simulink model "isi_corr"  (Simulink 7.0)
settings: Solver=ode45  StartTime=0.0  StopTime=500  RelTol=1e-3
undefined symbols (not defined in the file): bit_rev, pfb_coeff_gen_calc
libraries: xbsIndex_r4 x4180, casper_library x399, isi_corr_lib x8, xps_library x5, dspsigops x2, simulink x1
(part 1 of 45)
settings Model: Creator=wjm  LastModifiedBy=wjm

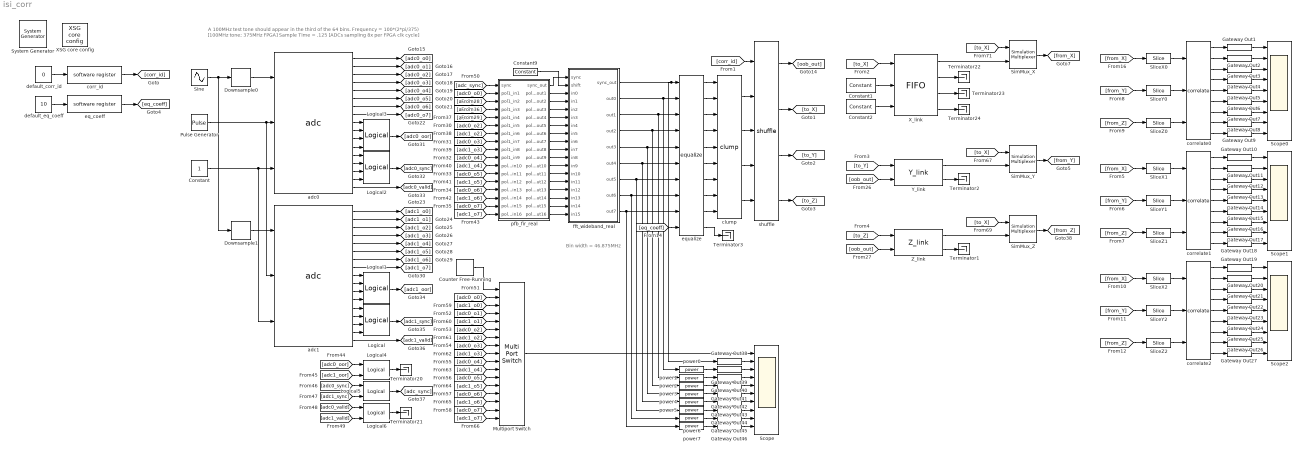
== system isi_corr ==
  note: A 100MHz test tone should appear in the third of the 64 bins.
      | Frequency = 100*(2*pi/375) [100MHz tone; 375MHz FPGA]
      | Sample Time = .125 [ADCs sampling 8x per FPGA clk cycle]
  note: Bin width = 46.875MHz
  " System Generator" : xbsIndex_r4/ System Generator  Tag=genX  SystemSampleTime=-1  xilinxfamily=virtex5  part=xc5vsx95t  speed=-1  package=ff1136  synthesis_tool=XST  clock_wrapper=Clock Enables  directory=./isi_corr/sysgen  testbench=off  simulink_period=1  sysclk_period=2.6667  dcm_input_clock_period=100  incr_netlist=off  trim_vbits=Everywhere in SubSystem  dbl_ovrd=According to Block Masks  core_generation=According to Block Masks  run_coregen=off  deprecated_control=off  eval_field=0  block_type=sysgen
      settings: block_version=10.1.1
  Constant : Constant  OutDataType=sfix(16)  OutScaling=2^0  SampleTime=1
  Constant1 : xbsIndex_r4/Constant  arith_type=Boolean  const=1  n_bits=1  bin_pt=0  explicit_period=on  period=1  equ=P=C  opselect=C  inp2=PCIN>>17  opr=+  inp1=P  carry=CIN  dbl_ovrd=off  block_type=constant
      settings: block_version=10.1.1
  Constant2 : xbsIndex_r4/Constant  arith_type=Boolean  const=1  n_bits=1  bin_pt=0  explicit_period=on  period=1  equ=P=C  opselect=C  inp2=PCIN>>17  opr=+  inp1=P  carry=CIN  dbl_ovrd=off  block_type=constant
      settings: block_version=10.1.1
  Constant9 : xbsIndex_r4/Constant  arith_type=Unsigned  const=127  n_bits=7  bin_pt=0  explicit_period=on  period=1  equ=P=C  opselect=C  inp2=PCIN>>17  opr=+  inp1=P  carry=CIN  dbl_ovrd=off  block_type=constant
      settings: block_version=10.1.1
  Counter Free-Running : simulink/Sources/Counter Free-Running  SystemSampleTime=-1  NumBits=4  tsamp=1/16
  Downsample0 : dspsigops/Downsample  N=2  phase=1  ic=0  smode=Allow multirate  fmode=Maintain input frame size
  Downsample1 : dspsigops/Downsample  N=2  phase=0  ic=0  smode=Allow multirate  fmode=Maintain input frame size
  Gateway Out{1..27,38..46} (36 alike) : xbsIndex_r4/Gateway Out  hdl_port=on  timing_constraint=None  locs_specified=off  LOCs={}  block_type=gatewayout
      settings: infoedit="Gateway out block.  Converts Xilinx fixed point inputs into ouputs of type Simulink integer, double, or fixed point.<P><P>Hardware notes:  In hardware these blocks become top level output ports or are discarded, depending on how they are configured."  block_version=10.1.3
  Logical{,1..3} (4 alike) : xbsIndex_r4/Logical  logical_function=OR  inputs=4  en=off  latency=0  precision=Full  arith_type=Unsigned  n_bits=1  bin_pt=0  align_bp=on  dbl_ovrd=off  block_type=logical
      settings: block_version=10.1.1
  Logical4 : xbsIndex_r4/Logical  logical_function=OR  inputs=2  en=off  latency=0  precision=Full  arith_type=Unsigned  n_bits=1  bin_pt=0  align_bp=on  dbl_ovrd=off  block_type=logical
      settings: block_version=10.1.1
  Logical5 : xbsIndex_r4/Logical  logical_function=OR  inputs=2  en=off  latency=0  precision=Full  arith_type=Unsigned  n_bits=1  bin_pt=0  align_bp=on  dbl_ovrd=off  block_type=logical
      settings: block_version=10.1.1
  Logical6 : xbsIndex_r4/Logical  logical_function=AND  inputs=2  en=off  latency=0  precision=Full  arith_type=Unsigned  n_bits=1  bin_pt=0  align_bp=on  dbl_ovrd=off  block_type=logical
      settings: block_version=10.1.1
  Multiport Switch : MultiPortSwitch  Inputs=16  zeroidx=on  OutDataType=sfix(16)  OutScaling=2^0
  Pulse Generator : DiscretePulseGenerator  Period=2^12
  Scope : Scope  NumInputPorts=10
      settings: YMin=-1~-1~-1~0~-8.13152e-020~0~0~0~0~0  YMax=1~1~1~0.00275~0.0014~0.00175~0.00175~0.00225~0.00175~0.00225  SaveName=ScopeData4
  Scope0 : Scope  NumInputPorts=9
      settings: YMin=-1~-1~-1~0~-8.13152e-020~0~0~0~0  YMax=1~1~1~0.00275~0.0014~0.00175~0.00175~0.00225~0.00175
  Scope1 : Scope  NumInputPorts=9
      settings: YMin=-1~-1~-1~0~-8.13152e-020~0~0~0~0  YMax=1~1~1~0.00275~0.0014~0.00175~0.00175~0.00225~0.00175
  Scope2 : Scope  NumInputPorts=9
      settings: YMin=-1~-1~-1~0~-8.13152e-020~0~0~0~0  YMax=1~1~1~0.00275~0.0014~0.00175~0.00175~0.00225~0.00175  SaveName=ScopeData2
  SimMux_X : xbsIndex_r4/Simulation Multiplexer  sim_sel=1  hw_sel=2  block_type=simmux
      settings: infoedit="Distinguish input subsystems as \"simulation only\" and \"generation only\".  The input specified For Simulation will be used during Simulink simulation.  The input specified For Generation will be used during code generation.  This block will typically be used on the output of a HW Co-Simulation, Black Box, or ModelSim subsystem.<P><P>Hardware Notes: This block costs nothing."  block_version=10.1.3
  SimMux_Y : xbsIndex_r4/Simulation Multiplexer  sim_sel=1  hw_sel=2  block_type=simmux
      settings: infoedit="Distinguish input subsystems as \"simulation only\" and \"generation only\".  The input specified For Simulation will be used during Simulink simulation.  The input specified For Generation will be used during code generation.  This block will typically be used on the output of a HW Co-Simulation, Black Box, or ModelSim subsystem.<P><P>Hardware Notes: This block costs nothing."  block_version=10.1.3
  SimMux_Z : xbsIndex_r4/Simulation Multiplexer  sim_sel=1  hw_sel=2  block_type=simmux
      settings: infoedit="Distinguish input subsystems as \"simulation only\" and \"generation only\".  The input specified For Simulation will be used during Simulink simulation.  The input specified For Generation will be used during code generation.  This block will typically be used on the output of a HW Co-Simulation, Black Box, or ModelSim subsystem.<P><P>Hardware Notes: This block costs nothing."  block_version=10.1.3
  Sine : Sin  Amplitude=.9  Frequency=100*(2*pi/375)  SampleTime=1/16
  SliceX0 : xbsIndex_r4/Slice  nbits=8  boolean_output=off  mode=Upper Bit Location + Width  bit1=0  base1=MSB of Input  bit0=0  base0=LSB of Input  dbl_ovrd=off  block_type=slice
      settings: infoedit="Extracts a given range of bits from each input sample and presents it at the output.  The output type is ordinarily unsigned with binary point at zero, but can be Boolean when the slice is one bit wide.<P><P>Hardware notes: In hardware this block costs nothing."  block_version=10.1.1
  SliceX1 : xbsIndex_r4/Slice  nbits=8  boolean_output=off  mode=Upper Bit Location + Width  bit1=-8  base1=MSB of Input  bit0=8  base0=LSB of Input  dbl_ovrd=off  block_type=slice
      settings: infoedit="Extracts a given range of bits from each input sample and presents it at the output.  The output type is ordinarily unsigned with binary point at zero, but can be Boolean when the slice is one bit wide.<P><P>Hardware notes: In hardware this block costs nothing."  block_version=10.1.1
  SliceX2 : xbsIndex_r4/Slice  nbits=8  boolean_output=off  mode=Upper Bit Location + Width  bit1=-16  base1=MSB of Input  bit0=16  base0=LSB of Input  dbl_ovrd=off  block_type=slice
      settings: infoedit="Extracts a given range of bits from each input sample and presents it at the output.  The output type is ordinarily unsigned with binary point at zero, but can be Boolean when the slice is one bit wide.<P><P>Hardware notes: In hardware this block costs nothing."  block_version=10.1.1
  SliceY0 : xbsIndex_r4/Slice  nbits=8  boolean_output=off  mode=Upper Bit Location + Width  bit1=0  base1=MSB of Input  bit0=0  base0=LSB of Input  dbl_ovrd=off  block_type=slice
      settings: infoedit="Extracts a given range of bits from each input sample and presents it at the output.  The output type is ordinarily unsigned with binary point at zero, but can be Boolean when the slice is one bit wide.<P><P>Hardware notes: In hardware this block costs nothing."  block_version=10.1.1
  SliceY1 : xbsIndex_r4/Slice  nbits=8  boolean_output=off  mode=Upper Bit Location + Width  bit1=-8  base1=MSB of Input  bit0=8  base0=LSB of Input  dbl_ovrd=off  block_type=slice
      settings: infoedit="Extracts a given range of bits from each input sample and presents it at the output.  The output type is ordinarily unsigned with binary point at zero, but can be Boolean when the slice is one bit wide.<P><P>Hardware notes: In hardware this block costs nothing."  block_version=10.1.1
  SliceY2 : xbsIndex_r4/Slice  nbits=8  boolean_output=off  mode=Upper Bit Location + Width  bit1=-16  base1=MSB of Input  bit0=16  base0=LSB of Input  dbl_ovrd=off  block_type=slice
      settings: infoedit="Extracts a given range of bits from each input sample and presents it at the output.  The output type is ordinarily unsigned with binary point at zero, but can be Boolean when the slice is one bit wide.<P><P>Hardware notes: In hardware this block costs nothing."  block_version=10.1.1
  SliceZ0 : xbsIndex_r4/Slice  nbits=8  boolean_output=off  mode=Upper Bit Location + Width  bit1=0  base1=MSB of Input  bit0=0  base0=LSB of Input  dbl_ovrd=off  block_type=slice
      settings: infoedit="Extracts a given range of bits from each input sample and presents it at the output.  The output type is ordinarily unsigned with binary point at zero, but can be Boolean when the slice is one bit wide.<P><P>Hardware notes: In hardware this block costs nothing."  block_version=10.1.1
  SliceZ1 : xbsIndex_r4/Slice  nbits=8  boolean_output=off  mode=Upper Bit Location + Width  bit1=-8  base1=MSB of Input  bit0=8  base0=LSB of Input  dbl_ovrd=off  block_type=slice
      settings: infoedit="Extracts a given range of bits from each input sample and presents it at the output.  The output type is ordinarily unsigned with binary point at zero, but can be Boolean when the slice is one bit wide.<P><P>Hardware notes: In hardware this block costs nothing."  block_version=10.1.1
  SliceZ2 : xbsIndex_r4/Slice  nbits=8  boolean_output=off  mode=Upper Bit Location + Width  bit1=-16  base1=MSB of Input  bit0=16  base0=LSB of Input  dbl_ovrd=off  block_type=slice
      settings: infoedit="Extracts a given range of bits from each input sample and presents it at the output.  The output type is ordinarily unsigned with binary point at zero, but can be Boolean when the slice is one bit wide.<P><P>Hardware notes: In hardware this block costs nothing."  block_version=10.1.1
  Terminator{1..3,20..24} (8 alike) : Terminator
  XSG core config : xps_library/XSG core config  Tag=xps:xsg  SystemSampleTime=-1  hw_sys=ROACH  ibob_linux=off  clk_src=adc0_clk  iBOB_clk_src=sys_clk  BEE2_ctrl_clk_src=sys_clk  BEE2_usr_clk_src=sys_clk  ROACH_clk_src=adc0_clk  CORR_clk_src=sys_clk  gpio_clk_io_group=iBOB:sma  gpio_clk_bit_index=0  clk_rate=375  sample_period=1  synthesis_tool=XST  mpc_type=powerpc440_ext
  X_link : xbsIndex_r4/FIFO  depth=16  percent_nbits=1  rst=off  en=off  use_dcount=off  use_almost_empty=off  almost_empty_thresh=2  use_almost_full=off  almost_full_thresh=14  dbl_ovrd=off  mem_type=Block RAM  block_type=fifo
      settings: block_version=10.1.1
  Y_link : isi_corr_lib/Y_link  SystemSampleTime=-1
      link override XAUI0/convert_tx_data: n_bits=32
      link override XAUI0/convert_tx_outofband: n_bits=4
      link override XAUI0/isi_corr_Y_link_XAUI0_rx_data: n_bits=32
      link override XAUI0/isi_corr_Y_link_XAUI0_rx_outofband: n_bits=4
      link override XAUI1/convert_tx_data: n_bits=32
      link override XAUI1/convert_tx_outofband: n_bits=4
      link override XAUI1/isi_corr_Y_link_XAUI1_rx_data: n_bits=32
      link override XAUI1/isi_corr_Y_link_XAUI1_rx_outofband: n_bits=4
  Z_link : isi_corr_lib/Z_link  SystemSampleTime=-1
      link override XAUI2/convert_tx_data: n_bits=32
      link override XAUI2/convert_tx_outofband: n_bits=4
      link override XAUI2/isi_corr_Z_link_XAUI2_rx_data: n_bits=32
      link override XAUI2/isi_corr_Z_link_XAUI2_rx_outofband: n_bits=4
      link override XAUI3/convert_tx_data: n_bits=32
      link override XAUI3/convert_tx_outofband: n_bits=4
      link override XAUI3/isi_corr_Z_link_XAUI3_rx_data: n_bits=32
      link override XAUI3/isi_corr_Z_link_XAUI3_rx_outofband: n_bits=4
  adc0 : xps_library/adc  Tag=xps:adc  SystemSampleTime=-1  adc_brd=adc0  adc_clk_rate=1500  adc_interleave=on  sample_period=1
  adc1 : xps_library/adc  Tag=xps:adc  SystemSampleTime=-1  adc_brd=adc1  adc_clk_rate=1500  adc_interleave=on  sample_period=1
  clump : isi_corr_lib/clump  SystemSampleTime=-1
  corr_id : xps_library/software register  Tag=xps:sw_reg  SystemSampleTime=-1  io_dir=From Processor  arith_type=Unsigned  bitwidth=32  bin_pt=0  sample_period=1  gw_name=isi_corr_corr_id_user_data_out
      link override corr_3ghz_10_1_corr_id_user_data_out: arith_type=Unsigned  n_bits=32  bin_pt=0  period=1
      link override corr_3ghz_10_1_exp_corr_id_user_data_out: arith_type=Unsigned  n_bits=32  bin_pt=0  period=1
      link override isi_corr_corr_id_user_data_out: arith_type=Unsigned  n_bits=32  bin_pt=0  period=1
  correlate0 : isi_corr_lib/correlate  SystemSampleTime=-1
  correlate1 : isi_corr_lib/correlate  SystemSampleTime=-1
  correlate2 : isi_corr_lib/correlate  SystemSampleTime=-1
  default_corr_id : Constant  Value=0  OutDataType=sfix(16)  OutScaling=2^0  SampleTime=1
  default_eq_coeff : Constant  Value=10  OutDataType=sfix(16)  OutScaling=2^0  SampleTime=1
  eq_coeff : xps_library/software register  Tag=xps:sw_reg  SystemSampleTime=-1  io_dir=From Processor  arith_type=Unsigned  bitwidth=32  bin_pt=0  sample_period=1  gw_name=isi_corr_eq_coeff_user_data_out
      link override corr_3ghz_10_1_corr_id_user_data_out: arith_type=Unsigned  n_bits=32  bin_pt=0  period=1
      link override corr_3ghz_10_1_exp_corr_id_user_data_out: arith_type=Unsigned  n_bits=32  bin_pt=0  period=1
      link override isi_corr_eq_coeff_user_data_out: arith_type=Unsigned  n_bits=32  bin_pt=0  period=1
  equalize : isi_corr_lib/equalize  SystemSampleTime=-1
  fft_wideband_real : Subsystem  AncestorBlock=casper_library/FFTs/fft_wideband_real  mask(fft_wideband_real){FFTSize=7, BitWidth=18, n_inputs=4, quantization=Truncate, overflow=Wrap, add_latency=2, mult_latency=2, bram_latency=3}
      mask prompts: FFTSize "Size of FFT: (2^?)"; BitWidth "Bit Width"; n_inputs "Number of Simultaneous Inputs: (2^?)"; quantization "Quantization Behavior"; overflow "Overflow Behavior"; add_latency "Add Latency"; mult_latency "Mult Latency"; bram_latency "BRAM Latency"
      mask init:
      | fft_wideband_real_init(gcb,...
      |     'FFTSize', FFTSize,...
      |     'BitWidth', BitWidth,...
      |     'n_inputs', n_inputs,...
      |     'quantization', quantization,...
      |     'overflow', overflow,...
      |     'add_latency', add_latency,...
      |     'mult_latency', mult_latency,...
      |     'bram_latency', bram_latency);
      settings: AttributesFormatString=FFTSize=7, n_inputs=4
      mask StyleString: edit,edit,edit,popup(Truncate|Round  (unbiased: +/- Inf)|Round  (unbiased: Even Values)),popup(Wrap|Saturate|Error),edit,edit,edit
  pfb_fir_real : Subsystem  AncestorBlock=casper_library/PFBs/pfb_fir_real  mask(pfb_fir_real){PFBSize=8, TotalTaps=4, WindowType=hamming, n_inputs=4, MakeBiplex=0, BitWidthIn=8, BitWidthOut=18, CoeffBitWidth=18, CoeffDistMem=0, add_latency=1, mult_latency=2, bram_latency=2, quantization=Truncate, fwidth=1}
      mask prompts: PFBSize "Size of PFB: (2^? pnts)"; TotalTaps "Total Number of Taps:"; WindowType "Windowing Function:"; n_inputs "Number of Simultaneous Inputs: (2^?)"; MakeBiplex "Make Biplex"; BitWidthIn "Input Bitwidth:"; BitWidthOut "Output Bitwidth:"; CoeffBitWidth "Coefficient Bitwidth:"; CoeffDistMem "Use Distributed Memory for Coeffs"; add_latency "Add Latency"; mult_latency "Mult Latency"; bram_latency "BRAM Latency"; quantization "Quantization Behavior"; fwidth "Bin Width Scaling (normal=1)"
      mask init:
      | pfb_fir_real_init(gcb,...
      |     'PFBSize', PFBSize,...
      |     'TotalTaps', TotalTaps,...
      |     'WindowType', WindowType,...
      |     'n_inputs', n_inputs,...
      |     'MakeBiplex', MakeBiplex,...
      |     'BitWidthIn', BitWidthIn,...
      |     'BitWidthOut', BitWidthOut,...
      |     'CoeffBitWidth', CoeffBitWidth,...
      |     'CoeffDistMem', CoeffDistMem,...
      |     'add_latency', add_latency,...
      |     'mult_latency', mult_latency,...
      |     'bram_latency', bram_latency,...
      |     'quantization', quantization,...
      |     'fwidth', fwidth);
      settings: AttributesFormatString=taps=4, add_latency=1
      mask Help: eval('xlWeb([getenv(''MLIB_ROOT''), ''\doc\htmlblocks\sp_pfb_fir_real\sp_pfb_fir_real.html''])')
      mask StyleString: edit,edit,popup(bartlett|barthannwin|blackman|blackmanharris|bohamwin|chebwin|flattopwin|gausswin|hamming|hann|kaiser|nuttallwin|parzenwin|rectwin|tukeywin|triang|userwindow),edit,edit,edit,edit,edit,edit,edit,edit,edit,popup(Truncate|Round  (unbiased: +/- Inf)|Round  (unbiased: Even Values)),edit
  power{0..7} (8 alike) : casper_library/Misc/power  SystemSampleTime=-1  BitWidth=18
  shuffle : isi_corr_lib/shuffle  SystemSampleTime=-1
  wires:
  adc1/9 -> Logical1/1
  adc1/10 -> Logical1/2
  adc1/11 -> Logical1/3
  adc1/12 -> Logical1/4
  adc1/13 -> Logical/1
  adc1/14 -> Logical/2
  adc1/15 -> Logical/3
  adc1/16 -> Logical/4
  Constant9/1 -> fft_wideband_real/2(shift)
  fft_wideband_real/2(out0) -> equalize/2, power0/1
  fft_wideband_real/3(out1) -> equalize/3, power1/1
  fft_wideband_real/4(out2) -> equalize/4, power2/1
  fft_wideband_real/5(out3) -> equalize/5, power3/1
  fft_wideband_real/6(out4) -> equalize/6, power4/1
  fft_wideband_real/7(out5) -> equalize/7, power5/1
  fft_wideband_real/8(out6) -> equalize/8, power6/1
  fft_wideband_real/9(out7) -> equalize/9, power7/1
  corr_id/1 -> [corr_id]
  default_corr_id/1 -> corr_id/1
  Z_link/1 -> SimMux_Z/2
  [from_X] -> SliceX0/1
  [from_Y] -> SliceY0/1
  [from_Z] -> SliceZ0/1
  fft_wideband_real/1(sync_out) -> equalize/1, Gateway Out38/1
  [from_X] -> SliceX1/1
  [from_Y] -> SliceY1/1
  [from_Z] -> SliceZ1/1
  [from_X] -> SliceX2/1
  [from_Y] -> SliceY2/1
  [from_Z] -> SliceZ2/1
  Gateway Out38/1 -> Scope/2
  Gateway Out39/1 -> Scope/3
  power0/1 -> Gateway Out39/1
  power1/1 -> Gateway Out40/1
  power2/1 -> Gateway Out41/1
  power3/1 -> Gateway Out42/1
  power4/1 -> Gateway Out43/1
  power5/1 -> Gateway Out44/1
  power6/1 -> Gateway Out45/1
  power7/1 -> Gateway Out46/1
  Gateway Out40/1 -> Scope/4
  Gateway Out41/1 -> Scope/5
  Gateway Out42/1 -> Scope/6
  Gateway Out43/1 -> Scope/7
  Gateway Out44/1 -> Scope/8
  Gateway Out45/1 -> Scope/9
  Gateway Out46/1 -> Scope/10
  adc0/9 -> Logical3/1
  adc0/10 -> Logical3/2
  adc0/11 -> Logical3/3
  adc0/12 -> Logical3/4
  adc0/1 -> [adc0_o0]
  adc0/2 -> [adc0_o1]
  adc0/3 -> [adc0_o2]
  adc0/4 -> [adc0_o3]
  adc0/5 -> [adc0_o4]
  adc0/6 -> [adc0_o5]
  adc0/7 -> [adc0_o6]
  adc0/8 -> [adc0_o7]
  adc1/1 -> [adc1_o0]
  adc1/2 -> [adc1_o1]
  adc1/3 -> [adc1_o2]
  adc1/4 -> [adc1_o3]
  adc1/5 -> [adc1_o4]
  adc1/6 -> [adc1_o5]
  adc1/7 -> [adc1_o6]
  adc1/8 -> [adc1_o7]
  Pulse Generator/1 -> adc0/2, adc1/2
  Constant/1 -> adc0/3, adc1/3
  Sine/1 -> Downsample0/1, Downsample1/1
  adc0/13 -> Logical2/1
  adc0/14 -> Logical2/2
  adc0/15 -> Logical2/3
  adc0/16 -> Logical2/4
  Logical2/1 -> [adc0_sync]
  adc0/17 -> [adc0_valid]
  Logical3/1 -> [adc0_oor]
  Logical1/1 -> [adc1_oor]
  adc1/17 -> [adc1_valid]
  Logical/1 -> [adc1_sync]
  [adc0_oor] -> Logical4/1
  [adc1_oor] -> Logical4/2
  [adc0_sync] -> Logical5/1
  [adc1_sync] -> Logical5/2
  [adc0_valid] -> Logical6/1
  [adc1_valid] -> Logical6/2
  Logical4/1 -> Terminator20/1
  Logical6/1 -> Terminator21/1
  Logical5/1 -> [adc_sync]
  Downsample0/1 -> adc0/1
  Downsample1/1 -> adc1/1
  [adc_sync] -> pfb_fir_real/1(sync)
  [adc0_o0] -> pfb_fir_real/2(pol1_in1)
  [adc1_o0] -> pfb_fir_real/3(pol1_in2)
  [adc0_o1] -> pfb_fir_real/4(pol1_in3)
  [adc1_o1] -> pfb_fir_real/5(pol1_in4)
  [adc0_o2] -> pfb_fir_real/6(pol1_in5)
  [adc1_o2] -> pfb_fir_real/7(pol1_in6)
  [adc0_o3] -> pfb_fir_real/8(pol1_in7)
  [adc1_o3] -> pfb_fir_real/9(pol1_in8)
  [adc0_o4] -> pfb_fir_real/10(pol1_in9)
  [adc1_o4] -> pfb_fir_real/11(pol1_in10)
  [adc0_o5] -> pfb_fir_real/12(pol1_in11)
  [adc1_o5] -> pfb_fir_real/13(pol1_in12)
  [adc0_o6] -> pfb_fir_real/14(pol1_in13)
  [adc1_o6] -> pfb_fir_real/15(pol1_in14)
  [adc0_o7] -> pfb_fir_real/16(pol1_in15)
  [adc1_o7] -> pfb_fir_real/17(pol1_in16)
  pfb_fir_real/1(sync_out) -> fft_wideband_real/1(sync)
  pfb_fir_real/2(pol1_out1) -> fft_wideband_real/3(in0)
  pfb_fir_real/3(pol1_out2) -> fft_wideband_real/4(in1)
  pfb_fir_real/4(pol1_out3) -> fft_wideband_real/5(in2)
  pfb_fir_real/5(pol1_out4) -> fft_wideband_real/6(in3)
  pfb_fir_real/6(pol1_out5) -> fft_wideband_real/7(in4)
  pfb_fir_real/7(pol1_out6) -> fft_wideband_real/8(in5)
  pfb_fir_real/8(pol1_out7) -> fft_wideband_real/9(in6)
  pfb_fir_real/9(pol1_out8) -> fft_wideband_real/10(in7)
  pfb_fir_real/10(pol1_out9) -> fft_wideband_real/11(in8)
  pfb_fir_real/11(pol1_out10) -> fft_wideband_real/12(in9)
  pfb_fir_real/12(pol1_out11) -> fft_wideband_real/13(in10)
  pfb_fir_real/13(pol1_out12) -> fft_wideband_real/14(in11)
  pfb_fir_real/14(pol1_out13) -> fft_wideband_real/15(in12)
  pfb_fir_real/15(pol1_out14) -> fft_wideband_real/16(in13)
  pfb_fir_real/16(pol1_out15) -> fft_wideband_real/17(in14)
  pfb_fir_real/17(pol1_out16) -> fft_wideband_real/18(in15)
  Counter Free-Running/1 -> Multiport Switch/1
  [adc0_o0] -> Multiport Switch/2
  [adc1_o0] -> Multiport Switch/3
  [adc0_o1] -> Multiport Switch/4
  [adc1_o1] -> Multiport Switch/5
  [adc0_o2] -> Multiport Switch/6
  [adc1_o2] -> Multiport Switch/7
  [adc0_o3] -> Multiport Switch/8
  [adc1_o3] -> Multiport Switch/9
  [adc0_o4] -> Multiport Switch/10
  [adc1_o4] -> Multiport Switch/11
  [adc0_o5] -> Multiport Switch/12
  [adc1_o5] -> Multiport Switch/13
  [adc0_o6] -> Multiport Switch/14
  [adc1_o6] -> Multiport Switch/15
  [adc0_o7] -> Multiport Switch/16
  SimMux_Y/1 -> [from_Y]
  [to_X] -> SimMux_Y/1
  SimMux_Z/1 -> [from_Z]
  [to_X] -> SimMux_Z/1
  [to_X] -> X_link/1
  Constant2/1 -> X_link/3
  X_link/1 -> SimMux_X/2
  X_link/2 -> Terminator22/1
  X_link/3 -> Terminator23/1
  X_link/4 -> Terminator24/1
  SimMux_X/1 -> [from_X]
  [to_X] -> SimMux_X/1
  equalize/1 -> clump/1
  equalize/2 -> clump/2
  equalize/3 -> clump/3
  equalize/4 -> clump/4
  equalize/5 -> clump/5
  equalize/6 -> clump/6
  equalize/7 -> clump/7
  equalize/8 -> clump/8
  equalize/9 -> clump/9
  clump/1 -> shuffle/2
  clump/2 -> shuffle/3
  clump/3 -> shuffle/4
  clump/4 -> shuffle/5
  shuffle/1 -> [oob_out]
  shuffle/2 -> [to_X]
  shuffle/3 -> [to_Y]
  shuffle/4 -> [to_Z]
  Constant1/1 -> X_link/2
  Y_link/1 -> SimMux_Y/2
  Z_link/2 -> Terminator1/1
  Y_link/2 -> Terminator2/1
  [to_Y] -> Y_link/1
  [oob_out] -> Y_link/2
  [to_Z] -> Z_link/1
  [oob_out] -> Z_link/2
  Gateway Out19/1 -> Scope2/1
  Gateway Out20/1 -> Scope2/2
  Gateway Out21/1 -> Scope2/3
  Gateway Out22/1 -> Scope2/4
  Gateway Out23/1 -> Scope2/5
  Gateway Out24/1 -> Scope2/6
  Gateway Out25/1 -> Scope2/7
  Gateway Out26/1 -> Scope2/8
  Gateway Out27/1 -> Scope2/9
  Gateway Out10/1 -> Scope1/1
  Gateway Out11/1 -> Scope1/2
  Gateway Out12/1 -> Scope1/3
  Gateway Out13/1 -> Scope1/4
  Gateway Out14/1 -> Scope1/5
  Gateway Out15/1 -> Scope1/6
  Gateway Out16/1 -> Scope1/7
  Gateway Out17/1 -> Scope1/8
  Gateway Out18/1 -> Scope1/9
  Gateway Out1/1 -> Scope0/1
  Gateway Out2/1 -> Scope0/2
  Gateway Out3/1 -> Scope0/3
  Gateway Out4/1 -> Scope0/4
  Gateway Out5/1 -> Scope0/5
  Gateway Out6/1 -> Scope0/6
  Gateway Out7/1 -> Scope0/7
  Gateway Out8/1 -> Scope0/8
  Gateway Out9/1 -> Scope0/9
  correlate0/1 -> Gateway Out1/1
  correlate0/2 -> Gateway Out2/1
  correlate0/3 -> Gateway Out3/1
  correlate0/4 -> Gateway Out4/1
  correlate0/5 -> Gateway Out5/1
  correlate0/6 -> Gateway Out6/1
  correlate0/7 -> Gateway Out7/1
  correlate0/8 -> Gateway Out8/1
  correlate0/9 -> Gateway Out9/1
  correlate1/1 -> Gateway Out10/1
  correlate1/2 -> Gateway Out11/1
  correlate1/3 -> Gateway Out12/1
  correlate1/4 -> Gateway Out13/1
  correlate1/5 -> Gateway Out14/1
  correlate1/6 -> Gateway Out15/1
  correlate1/7 -> Gateway Out16/1
  correlate1/8 -> Gateway Out17/1
  correlate1/9 -> Gateway Out18/1
  correlate2/1 -> Gateway Out19/1
  correlate2/2 -> Gateway Out20/1
  correlate2/3 -> Gateway Out21/1
  correlate2/4 -> Gateway Out22/1
  correlate2/5 -> Gateway Out23/1
  correlate2/6 -> Gateway Out24/1
  correlate2/7 -> Gateway Out25/1
  correlate2/8 -> Gateway Out26/1
  correlate2/9 -> Gateway Out27/1
  equalize/10 -> Terminator3/1
  eq_coeff/1 -> [eq_coeff]
  default_eq_coeff/1 -> eq_coeff/1
  [eq_coeff] -> equalize/10
  [corr_id] -> shuffle/1
  [adc1_o7] -> Multiport Switch/17
  Multiport Switch/1 -> Scope/1
  SliceX0/1 -> correlate0/1
  SliceY0/1 -> correlate0/2
  SliceZ0/1 -> correlate0/3
  SliceX1/1 -> correlate1/1
  SliceY1/1 -> correlate1/2
  SliceZ1/1 -> correlate1/3
  SliceX2/1 -> correlate2/1
  SliceY2/1 -> correlate2/2
  SliceZ2/1 -> correlate2/3

== system isi_corr/fft_wideband_real ==
  sync : Inport  OutDataType=sfix(16)  OutScaling=2^0
  shift : Inport  Port=2  OutDataType=sfix(16)  OutScaling=2^0
  in0 : Inport  Port=3  OutDataType=sfix(16)  OutScaling=2^0
  in1 : Inport  Port=4  OutDataType=sfix(16)  OutScaling=2^0
  in2 : Inport  Port=5  OutDataType=sfix(16)  OutScaling=2^0
  in3 : Inport  Port=6  OutDataType=sfix(16)  OutScaling=2^0
  in4 : Inport  Port=7
  in5 : Inport  Port=8
  in6 : Inport  Port=9
  in7 : Inport  Port=10
  in8 : Inport  Port=11
  in9 : Inport  Port=12
  in10 : Inport  Port=13
  in11 : Inport  Port=14
  in12 : Inport  Port=15
  in13 : Inport  Port=16
  in14 : Inport  Port=17
  in15 : Inport  Port=18
  fft_biplex_real_4x0 : Subsystem  AncestorBlock=casper_library/FFTs/fft_biplex_real_4x  mask(fft_biplex_real_4x){FFTSize=3, BitWidth=18, quantization=Truncate, overflow=Wrap, add_latency=2, mult_latency=2, bram_latency=3}
      mask prompts: FFTSize "Size of FFT: (2^? pnts)"; BitWidth "Bitwidth: (Max Efficiency at 18 bits)"; quantization "Quantization Behavior"; overflow "Overflow Behavior"; add_latency "Add Latency"; mult_latency "Mult Latency"; bram_latency "BRAM Latency"
      mask init:
      | fft_biplex_real_4x_init(gcb,...
      |     'FFTSize', FFTSize,...
      |     'BitWidth', BitWidth,...
      |     'quantization', quantization,...
      |     'overflow', overflow,...
      |     'add_latency', add_latency,...
      |     'mult_latency', mult_latency,...
      |     'bram_latency', bram_latency);
      settings: AttributesFormatString=FFTSize=3, BitWidth=18
      mask StyleString: edit,edit,popup(Truncate|Round  (unbiased: +/- Inf)|Round  (unbiased: Even Values)),popup(Wrap|Saturate|Error),edit,edit,edit
  fft_biplex_real_4x1 : Subsystem  AncestorBlock=casper_library/FFTs/fft_biplex_real_4x  mask(fft_biplex_real_4x){FFTSize=3, BitWidth=18, quantization=Truncate, overflow=Wrap, add_latency=2, mult_latency=2, bram_latency=3}
      mask prompts: FFTSize "Size of FFT: (2^? pnts)"; BitWidth "Bitwidth: (Max Efficiency at 18 bits)"; quantization "Quantization Behavior"; overflow "Overflow Behavior"; add_latency "Add Latency"; mult_latency "Mult Latency"; bram_latency "BRAM Latency"
      mask init: same as isi_corr/fft_wideband_real/fft_biplex_real_4x0
      settings: AttributesFormatString=FFTSize=3, BitWidth=18
      mask StyleString: edit,edit,popup(Truncate|Round  (unbiased: +/- Inf)|Round  (unbiased: Even Values)),popup(Wrap|Saturate|Error),edit,edit,edit
  fft_biplex_real_4x2 : Subsystem  AncestorBlock=casper_library/FFTs/fft_biplex_real_4x  mask(fft_biplex_real_4x){FFTSize=3, BitWidth=18, quantization=Truncate, overflow=Wrap, add_latency=2, mult_latency=2, bram_latency=3}
      mask prompts: FFTSize "Size of FFT: (2^? pnts)"; BitWidth "Bitwidth: (Max Efficiency at 18 bits)"; quantization "Quantization Behavior"; overflow "Overflow Behavior"; add_latency "Add Latency"; mult_latency "Mult Latency"; bram_latency "BRAM Latency"
      mask init: same as isi_corr/fft_wideband_real/fft_biplex_real_4x0
      settings: AttributesFormatString=FFTSize=3, BitWidth=18
      mask StyleString: edit,edit,popup(Truncate|Round  (unbiased: +/- Inf)|Round  (unbiased: Even Values)),popup(Wrap|Saturate|Error),edit,edit,edit
  fft_biplex_real_4x3 : Subsystem  AncestorBlock=casper_library/FFTs/fft_biplex_real_4x  mask(fft_biplex_real_4x){FFTSize=3, BitWidth=18, quantization=Truncate, overflow=Wrap, add_latency=2, mult_latency=2, bram_latency=3}
      mask prompts: FFTSize "Size of FFT: (2^? pnts)"; BitWidth "Bitwidth: (Max Efficiency at 18 bits)"; quantization "Quantization Behavior"; overflow "Overflow Behavior"; add_latency "Add Latency"; mult_latency "Mult Latency"; bram_latency "BRAM Latency"
      mask init: same as isi_corr/fft_wideband_real/fft_biplex_real_4x0
      settings: AttributesFormatString=FFTSize=3, BitWidth=18
      mask StyleString: edit,edit,popup(Truncate|Round  (unbiased: +/- Inf)|Round  (unbiased: Even Values)),popup(Wrap|Saturate|Error),edit,edit,edit
  fft_direct : Subsystem  AncestorBlock=casper_library/FFTs/fft_direct  mask(fft_direct){FFTSize=4, BitWidth=18, add_latency=2, mult_latency=2, bram_latency=3, quantization=Truncate, overflow=Wrap, MapTail=1, LargerFFTSize=7, StartStage=4}
      mask prompts: FFTSize "Size of FFT: (2^?)"; BitWidth "Bit Width"; add_latency "Add Latency"; mult_latency "Mult Latency"; bram_latency "BRAM Latency"; quantization "Quantization Behavior"; overflow "Overflow Behavior"; MapTail "Map Tail of Larger FFT"; LargerFFTSize "Size of Larger FFT (2^?):"; StartStage "Start Stage of Map Tail:"
      mask init:
      | fft_direct_init(gcb,...
      |     'FFTSize', FFTSize,...
      |     'BitWidth', BitWidth,...
      |     'add_latency', add_latency,...
      |     'mult_latency', mult_latency,...
      |     'bram_latency', bram_latency,...
      |     'quantization', quantization,...
      |     'overflow', overflow,...
      |     'MapTail', MapTail,...
      |     'LargerFFTSize', LargerFFTSize,...
      |     'StartStage', StartStage);
      settings: AttributesFormatString=FFTSize=4
      mask StyleString: edit,edit,edit,edit,edit,popup(Truncate|Round  (unbiased: +/- Inf)|Round  (unbiased: Even Values)),popup(Wrap|Saturate|Error),edit,edit,edit
  fft_unscrambler : Subsystem  AncestorBlock=casper_library/FFTs/fft_unscrambler  mask(fft_unscrambler){FFTSize=6, n_inputs=3, bram_latency=3}
      mask prompts: FFTSize "Size of FFT: (2^?)"; n_inputs "Number of Simultaneous Inputs: (2^?)"; bram_latency "BRAM Latency"
      mask init:
      | fft_unscrambler_init(gcb,...
      |     'FFTSize',FFTSize,... 
      |     'n_inputs', n_inputs,...
      |     'bram_latency', bram_latency);
      settings: AttributesFormatString=FFTSize=6, n_inputs=3
  slice : xbsIndex_r4/Slice  nbits=4  boolean_output=off  mode=Lower Bit Location + Width  bit1=0  base1=MSB of Input  bit0=3  base0=LSB of Input  dbl_ovrd=off  block_type=slice
      settings: infoedit="Extracts a given range of bits from each input sample and presents it at the output.  The output type is ordinarily unsigned with binary point at zero, but can be Boolean when the slice is one bit wide.<P><P>Hardware notes: In hardware this block costs nothing."  block_version=9.1.01
  sync_out : Outport
  out0 : Outport  Port=2
  out1 : Outport  Port=3
  out2 : Outport  Port=4
  out3 : Outport  Port=5
  out4 : Outport  Port=6
  out5 : Outport  Port=7
  out6 : Outport  Port=8
  out7 : Outport  Port=9
  wires:
  in0/1 -> fft_biplex_real_4x0/1(pol1)
  in1/1 -> fft_biplex_real_4x0/2(pol2)
  in2/1 -> fft_biplex_real_4x0/3(pol3)
  in3/1 -> fft_biplex_real_4x0/4(pol4)
  shift/1 -> fft_biplex_real_4x0/6(shift), fft_biplex_real_4x1/6(shift), fft_biplex_real_4x2/6(shift), fft_biplex_real_4x3/6(shift), slice/1
  sync/1 -> fft_biplex_real_4x0/5(sync), fft_biplex_real_4x1/5(sync), fft_biplex_real_4x2/5(sync), fft_biplex_real_4x3/5(sync)
  in4/1 -> fft_biplex_real_4x1/1(pol1)
  in5/1 -> fft_biplex_real_4x1/2(pol2)
  in6/1 -> fft_biplex_real_4x1/3(pol3)
  in7/1 -> fft_biplex_real_4x1/4(pol4)
  in8/1 -> fft_biplex_real_4x2/1(pol1)
  in9/1 -> fft_biplex_real_4x2/2(pol2)
  in10/1 -> fft_biplex_real_4x2/3(pol3)
  in11/1 -> fft_biplex_real_4x2/4(pol4)
  in12/1 -> fft_biplex_real_4x3/1(pol1)
  in13/1 -> fft_biplex_real_4x3/2(pol2)
  in14/1 -> fft_biplex_real_4x3/3(pol3)
  in15/1 -> fft_biplex_real_4x3/4(pol4)
  slice/1 -> fft_direct/2(shift)
  fft_biplex_real_4x0/6(sync_out) -> fft_direct/1(sync)
  fft_biplex_real_4x0/1(pol1_out) -> fft_direct/3(in0)
  fft_biplex_real_4x0/2(pol2_out) -> fft_direct/4(in1)
  fft_biplex_real_4x0/3(pol3_out) -> fft_direct/5(in2)
  fft_biplex_real_4x0/4(pol4_out) -> fft_direct/6(in3)
  fft_biplex_real_4x1/1(pol1_out) -> fft_direct/7(in4)
  fft_biplex_real_4x1/2(pol2_out) -> fft_direct/8(in5)
  fft_biplex_real_4x1/3(pol3_out) -> fft_direct/9(in6)
  fft_biplex_real_4x1/4(pol4_out) -> fft_direct/10(in7)
  fft_biplex_real_4x2/1(pol1_out) -> fft_direct/11(in8)
  fft_biplex_real_4x2/2(pol2_out) -> fft_direct/12(in9)
  fft_biplex_real_4x2/3(pol3_out) -> fft_direct/13(in10)
  fft_biplex_real_4x2/4(pol4_out) -> fft_direct/14(in11)
  fft_biplex_real_4x3/1(pol1_out) -> fft_direct/15(in12)
  fft_biplex_real_4x3/2(pol2_out) -> fft_direct/16(in13)
  fft_biplex_real_4x3/3(pol3_out) -> fft_direct/17(in14)
  fft_biplex_real_4x3/4(pol4_out) -> fft_direct/18(in15)
  fft_direct/1(sync_out) -> fft_unscrambler/1(sync)
  fft_unscrambler/1(sync_out) -> sync_out/1
  fft_direct/2(out0) -> fft_unscrambler/2(In1)
  fft_unscrambler/2(Out1) -> out0/1
  fft_direct/3(out1) -> fft_unscrambler/3(In2)
  fft_unscrambler/3(Out2) -> out1/1
  fft_direct/4(out2) -> fft_unscrambler/4(In3)
  fft_unscrambler/4(Out3) -> out2/1
  fft_direct/5(out3) -> fft_unscrambler/5(In4)
  fft_unscrambler/5(Out4) -> out3/1
  fft_direct/6(out4) -> fft_unscrambler/6(In5)
  fft_unscrambler/6(Out5) -> out4/1
  fft_direct/7(out5) -> fft_unscrambler/7(In6)
  fft_unscrambler/7(Out6) -> out5/1
  fft_direct/8(out6) -> fft_unscrambler/8(In7)
  fft_unscrambler/8(Out7) -> out6/1
  fft_direct/9(out7) -> fft_unscrambler/9(In8)
  fft_unscrambler/9(Out8) -> out7/1
